simulink model "isi_correlator_tvg"  (Simulink 7.3)
settings: Solver=ode45  StartTime=0.0  StopTime=10.0  RelTol=1e-3
undefined symbols (not defined in the file or in its folder): pfb_coeff_gen_calc, ROACH
libraries: xbsIndex_r4 x5206, casper_library x454, isi_correlator_lib x28, dspsigops x16, xps_library x14
(part 1 of 51)
settings Model: Creator=wjm  LastModifiedBy=wjm

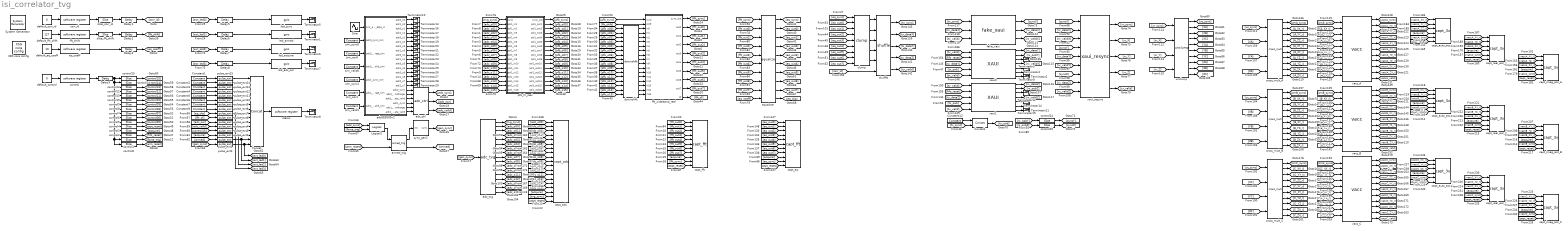
== system isi_correlator_tvg ==
  " System Generator" : xbsIndex_r4/ System Generator  Tag=genX  SystemSampleTime=-1  xilinxfamily=virtex5  part=xc5vsx95t  speed=-1  package=ff1136  synthesis_tool=XST  clock_wrapper=Clock Enables  directory=./isi_correlator_tvg/sysgen  testbench=off  simulink_period=1  sysclk_period=5  dcm_input_clock_period=10  incr_netlist=off  trim_vbits=Everywhere in SubSystem  dbl_ovrd=According to Block Masks  core_generation=According to Block Masks  run_coregen=off  deprecated_control=off  eval_field=0  block_type=sysgen
      settings: block_version=11.3
  Concat : xbsIndex_r4/Concat  num_inputs=16  dbl_ovrd=off  block_type=concat
      settings: block_version=10.1.3
  Concat1 : xbsIndex_r4/Concat  num_inputs=2  dbl_ovrd=off  block_type=concat
      settings: block_version=11.3
  Constant{1..7,9} (8 alike) : xbsIndex_r4/Constant  arith_type=Boolean  const=0  n_bits=1  bin_pt=0  explicit_period=on  period=1  equ=P=C  opselect=C  inp2=PCIN>>17  opr=+  inp1=P  carry=CIN  dbl_ovrd=off  block_type=constant
      settings: block_version=10.1.1
  Constant12 : xbsIndex_r4/Constant  arith_type=Unsigned  const=0  n_bits=3  bin_pt=0  explicit_period=on  period=1  equ=P=C  opselect=C  inp2=PCIN>>17  opr=+  inp1=P  carry=CIN  dbl_ovrd=off  block_type=constant
      settings: block_version=10.1.1
  Delay{1..3,9} (4 alike) : xbsIndex_r4/Delay  en=off  latency=8  dbl_ovrd=off  reg_retiming=off  block_type=delay
  Delay{5..8} (4 alike) : xbsIndex_r4/Delay  en=off  latency=3  dbl_ovrd=off  reg_retiming=off  block_type=delay
  Logical1 : xbsIndex_r4/Logical  logical_function=OR  inputs=2  en=off  latency=1  precision=Full  arith_type=Unsigned  n_bits=1  bin_pt=0  align_bp=on  dbl_ovrd=off  block_type=logical
      settings: block_version=10.1.1
  Sine : Sin  Amplitude=.9  Bias=0  Frequency=37.5*(2*pi/200)  Phase=0  Samples=10  SampleTime=1/16  Offset=0
  Terminator{1..29} (29 alike) : Terminator
  XSG core config : xps_library/XSG core config  Tag=xps:xsg  SystemSampleTime=-1  hw_sys=ROACH:sx95t  ibob_linux=off  clk_src=adc0_clk  iBOB_clk_src=sys_clk  BEE2_ctrl_clk_src=sys_clk  BEE2_usr_clk_src=sys_clk  ROACH_clk_src=adc0_clk  CORR_clk_src=sys_clk  gpio_clk_io_group=iBOB:sma  gpio_clk_bit_index=0  clk_rate=200  sample_period=1  synthesis_tool=XST  mpc_type=powerpc440_ext
  adc083000x2 : Subsystem  Tag=xps:adc083000x2  AncestorBlock=xps_library/adc083000x2  mask(adc_083000x2){use_adc0=on, use_adc1=on, demux_adc0=off, clock_sync=on, verbose=off, adc_clk_rate=800, sample_period=1}
      mask prompts: use_adc0 "Use ZD0K0?"; use_adc1 "Use ZDOK1?"; demux_adc0 "Demux outputs of adc0?"; clock_sync "Interleave 2 ADC boards?"; verbose "Verbose Block Creation?"; adc_clk_rate "ADC clock rate (MHz)"; sample_period "Sample Period"
      mask help: The ADC block converts analog inputs to digital outputs. Every clock cycle, the inputs are sampled and digitized to 8 bit binary point numbers in the range of [-1, 1) and are then output by the adc.
      mask init:
      | adc083000_mask;
      mask Help: eval('xlWeb([getenv(''MLIB_ROOT''), ''\doc\html\sys_adc\sys_adc.html''])')
  adc_ctrl : isi_correlator_lib/adc_ctrl  SystemSampleTime=-1
  adc_tvg : isi_correlator_lib/adc_tvg  SystemSampleTime=-1
  armed_trig : isi_correlator_lib/armed_trig  SystemSampleTime=-1
  capt_adc : isi_correlator_lib/capt_adc  SystemSampleTime=-1
  capt_auto_acc_A : isi_correlator_lib/capt_3x  SystemSampleTime=-1
  capt_auto_acc_B : isi_correlator_lib/capt_3x  SystemSampleTime=-1
  capt_auto_acc_C : isi_correlator_lib/capt_3x  SystemSampleTime=-1
  capt_eq : isi_correlator_lib/capt_fft  SystemSampleTime=-1  bit_width=4
  capt_fft : isi_correlator_lib/capt_fft  SystemSampleTime=-1  bit_width=18
  capt_imag_acc_A : isi_correlator_lib/capt_3x  SystemSampleTime=-1
  capt_imag_acc_B : isi_correlator_lib/capt_3x  SystemSampleTime=-1
  capt_imag_acc_C : isi_correlator_lib/capt_3x  SystemSampleTime=-1
  capt_real_acc_A : isi_correlator_lib/capt_3x  SystemSampleTime=-1
  capt_real_acc_B : isi_correlator_lib/capt_3x  SystemSampleTime=-1
  capt_real_acc_C : isi_correlator_lib/capt_3x  SystemSampleTime=-1
  clump : isi_correlator_lib/clump  SystemSampleTime=-1  latency=1
  control : xps_library/software register  Tag=xps:sw_reg  SystemSampleTime=-1  io_dir=From Processor  arith_type=Unsigned  bitwidth=32  bin_pt=0  sample_period=1  gw_name=isi_correlator_tvg_control_user_data_out
      link override corr_3ghz_10_1_corr_id_user_data_out: arith_type=Unsigned  n_bits=32  bin_pt=0  period=1
      link override corr_3ghz_10_1_exp_corr_id_user_data_out: arith_type=Unsigned  n_bits=32  bin_pt=0  period=1
      link override isi_corr_eq_coeff_user_data_out: arith_type=Unsigned  n_bits=32  bin_pt=0  period=1
      link override isi_correlator_control_user_data_out: arith_type=Unsigned  n_bits=32  bin_pt=0  period=1
      link override isi_correlator_cropped_control_user_data_out: arith_type=Unsigned  n_bits=32  bin_pt=0  period=1
      link override isi_correlator_eq_coeff_user_data_out: arith_type=Unsigned  n_bits=32  bin_pt=0  period=1
      link override isi_spectrometer_control_user_data_out: arith_type=Unsigned  n_bits=32  bin_pt=0  period=1
      link override isi_spectrometer_cropped_control_user_data_out: arith_type=Unsigned  n_bits=32  bin_pt=0  period=1
      link override isi_spectrometer_with_pfb_control_user_data_out: arith_type=Unsigned  n_bits=32  bin_pt=0  period=1
  control0 : xbsIndex_r4/Slice  nbits=1  boolean_output=on  mode=Lower Bit Location + Width  bit1=0  base1=MSB of Input  bit0=0  base0=LSB of Input  dbl_ovrd=off  block_type=slice
      settings: infoedit="Extracts a given range of bits from each input sample and presents it at the output.  The output type is ordinarily unsigned with binary point at zero, but can be Boolean when the slice is one bit wide.<P><P>Hardware notes: In hardware this block costs nothing."  block_version=10.1.3
  control1 : xbsIndex_r4/Slice  nbits=1  boolean_output=on  mode=Lower Bit Location + Width  bit1=0  base1=MSB of Input  bit0=1  base0=LSB of Input  dbl_ovrd=off  block_type=slice
      settings: infoedit="Extracts a given range of bits from each input sample and presents it at the output.  The output type is ordinarily unsigned with binary point at zero, but can be Boolean when the slice is one bit wide.<P><P>Hardware notes: In hardware this block costs nothing."  block_version=10.1.3
  control10 : xbsIndex_r4/Slice  nbits=1  boolean_output=on  mode=Lower Bit Location + Width  bit1=0  base1=MSB of Input  bit0=10  base0=LSB of Input  dbl_ovrd=off  block_type=slice
      settings: infoedit="Extracts a given range of bits from each input sample and presents it at the output.  The output type is ordinarily unsigned with binary point at zero, but can be Boolean when the slice is one bit wide.<P><P>Hardware notes: In hardware this block costs nothing."  block_version=10.1.3
  control11 : xbsIndex_r4/Slice  nbits=1  boolean_output=on  mode=Lower Bit Location + Width  bit1=0  base1=MSB of Input  bit0=11  base0=LSB of Input  dbl_ovrd=off  block_type=slice
      settings: infoedit="Extracts a given range of bits from each input sample and presents it at the output.  The output type is ordinarily unsigned with binary point at zero, but can be Boolean when the slice is one bit wide.<P><P>Hardware notes: In hardware this block costs nothing."  block_version=10.1.3
  control12 : xbsIndex_r4/Slice  nbits=1  boolean_output=on  mode=Lower Bit Location + Width  bit1=0  base1=MSB of Input  bit0=12  base0=LSB of Input  dbl_ovrd=off  block_type=slice
      settings: infoedit="Extracts a given range of bits from each input sample and presents it at the output.  The output type is ordinarily unsigned with binary point at zero, but can be Boolean when the slice is one bit wide.<P><P>Hardware notes: In hardware this block costs nothing."  block_version=10.1.3
  control13 : xbsIndex_r4/Slice  nbits=1  boolean_output=on  mode=Lower Bit Location + Width  bit1=0  base1=MSB of Input  bit0=13  base0=LSB of Input  dbl_ovrd=off  block_type=slice
      settings: infoedit="Extracts a given range of bits from each input sample and presents it at the output.  The output type is ordinarily unsigned with binary point at zero, but can be Boolean when the slice is one bit wide.<P><P>Hardware notes: In hardware this block costs nothing."  block_version=10.1.3
  control14 : xbsIndex_r4/Slice  nbits=1  boolean_output=on  mode=Lower Bit Location + Width  bit1=0  base1=MSB of Input  bit0=14  base0=LSB of Input  dbl_ovrd=off  block_type=slice
      settings: infoedit="Extracts a given range of bits from each input sample and presents it at the output.  The output type is ordinarily unsigned with binary point at zero, but can be Boolean when the slice is one bit wide.<P><P>Hardware notes: In hardware this block costs nothing."  block_version=10.1.3
  control15 : xbsIndex_r4/Slice  nbits=1  boolean_output=on  mode=Lower Bit Location + Width  bit1=0  base1=MSB of Input  bit0=15  base0=LSB of Input  dbl_ovrd=off  block_type=slice
      settings: infoedit="Extracts a given range of bits from each input sample and presents it at the output.  The output type is ordinarily unsigned with binary point at zero, but can be Boolean when the slice is one bit wide.<P><P>Hardware notes: In hardware this block costs nothing."  block_version=10.1.3
  control2 : xbsIndex_r4/Slice  nbits=1  boolean_output=on  mode=Lower Bit Location + Width  bit1=0  base1=MSB of Input  bit0=2  base0=LSB of Input  dbl_ovrd=off  block_type=slice
      settings: infoedit="Extracts a given range of bits from each input sample and presents it at the output.  The output type is ordinarily unsigned with binary point at zero, but can be Boolean when the slice is one bit wide.<P><P>Hardware notes: In hardware this block costs nothing."  block_version=10.1.3
  control24 : xbsIndex_r4/Slice  nbits=1  boolean_output=on  mode=Lower Bit Location + Width  bit1=0  base1=MSB of Input  bit0=0  base0=LSB of Input  dbl_ovrd=off  block_type=slice
      settings: infoedit="Extracts a given range of bits from each input sample and presents it at the output.  The output type is ordinarily unsigned with binary point at zero, but can be Boolean when the slice is one bit wide.<P><P>Hardware notes: In hardware this block costs nothing."  block_version=10.1.3
  control25 : xbsIndex_r4/Slice  nbits=1  boolean_output=on  mode=Lower Bit Location + Width  bit1=0  base1=MSB of Input  bit0=0  base0=LSB of Input  dbl_ovrd=off  block_type=slice
      settings: infoedit="Extracts a given range of bits from each input sample and presents it at the output.  The output type is ordinarily unsigned with binary point at zero, but can be Boolean when the slice is one bit wide.<P><P>Hardware notes: In hardware this block costs nothing."  block_version=10.1.3
  control3 : xbsIndex_r4/Slice  nbits=1  boolean_output=on  mode=Lower Bit Location + Width  bit1=0  base1=MSB of Input  bit0=3  base0=LSB of Input  dbl_ovrd=off  block_type=slice
      settings: infoedit="Extracts a given range of bits from each input sample and presents it at the output.  The output type is ordinarily unsigned with binary point at zero, but can be Boolean when the slice is one bit wide.<P><P>Hardware notes: In hardware this block costs nothing."  block_version=10.1.3
  control4 : xbsIndex_r4/Slice  nbits=1  boolean_output=on  mode=Lower Bit Location + Width  bit1=0  base1=MSB of Input  bit0=4  base0=LSB of Input  dbl_ovrd=off  block_type=slice
      settings: infoedit="Extracts a given range of bits from each input sample and presents it at the output.  The output type is ordinarily unsigned with binary point at zero, but can be Boolean when the slice is one bit wide.<P><P>Hardware notes: In hardware this block costs nothing."  block_version=10.1.3
  control5 : xbsIndex_r4/Slice  nbits=1  boolean_output=on  mode=Lower Bit Location + Width  bit1=0  base1=MSB of Input  bit0=5  base0=LSB of Input  dbl_ovrd=off  block_type=slice
      settings: infoedit="Extracts a given range of bits from each input sample and presents it at the output.  The output type is ordinarily unsigned with binary point at zero, but can be Boolean when the slice is one bit wide.<P><P>Hardware notes: In hardware this block costs nothing."  block_version=10.1.3
  control6 : xbsIndex_r4/Slice  nbits=1  boolean_output=on  mode=Lower Bit Location + Width  bit1=0  base1=MSB of Input  bit0=6  base0=LSB of Input  dbl_ovrd=off  block_type=slice
      settings: infoedit="Extracts a given range of bits from each input sample and presents it at the output.  The output type is ordinarily unsigned with binary point at zero, but can be Boolean when the slice is one bit wide.<P><P>Hardware notes: In hardware this block costs nothing."  block_version=10.1.3
  control7 : xbsIndex_r4/Slice  nbits=1  boolean_output=on  mode=Lower Bit Location + Width  bit1=0  base1=MSB of Input  bit0=7  base0=LSB of Input  dbl_ovrd=off  block_type=slice
      settings: infoedit="Extracts a given range of bits from each input sample and presents it at the output.  The output type is ordinarily unsigned with binary point at zero, but can be Boolean when the slice is one bit wide.<P><P>Hardware notes: In hardware this block costs nothing."  block_version=10.1.3
  control8 : xbsIndex_r4/Slice  nbits=1  boolean_output=on  mode=Lower Bit Location + Width  bit1=0  base1=MSB of Input  bit0=8  base0=LSB of Input  dbl_ovrd=off  block_type=slice
      settings: infoedit="Extracts a given range of bits from each input sample and presents it at the output.  The output type is ordinarily unsigned with binary point at zero, but can be Boolean when the slice is one bit wide.<P><P>Hardware notes: In hardware this block costs nothing."  block_version=10.1.3
  control9 : xbsIndex_r4/Slice  nbits=1  boolean_output=on  mode=Lower Bit Location + Width  bit1=0  base1=MSB of Input  bit0=9  base0=LSB of Input  dbl_ovrd=off  block_type=slice
      settings: infoedit="Extracts a given range of bits from each input sample and presents it at the output.  The output type is ordinarily unsigned with binary point at zero, but can be Boolean when the slice is one bit wide.<P><P>Hardware notes: In hardware this block costs nothing."  block_version=10.1.3
  corr_id : xps_library/software register  Tag=xps:sw_reg  SystemSampleTime=-1  io_dir=From Processor  arith_type=Unsigned  bitwidth=32  bin_pt=0  sample_period=1  gw_name=isi_correlator_tvg_corr_id_user_data_out
      link override corr_3ghz_10_1_corr_id_user_data_out: arith_type=Unsigned  n_bits=32  bin_pt=0  period=1
      link override corr_3ghz_10_1_exp_corr_id_user_data_out: arith_type=Unsigned  n_bits=32  bin_pt=0  period=1
      link override isi_corr_corr_id_user_data_out: arith_type=Unsigned  n_bits=32  bin_pt=0  period=1
      link override isi_correlator_corr_id_user_data_out: arith_type=Unsigned  n_bits=32  bin_pt=0  period=1
      link override isi_correlator_cropped_corr_id_user_data_out: arith_type=Unsigned  n_bits=32  bin_pt=0  period=1
      link override isi_spectrometer_corr_id_user_data_out: arith_type=Unsigned  n_bits=32  bin_pt=0  period=1
      link override isi_spectrometer_cropped_corr_id_user_data_out: arith_type=Unsigned  n_bits=32  bin_pt=0  period=1
      link override isi_spectrometer_with_pfb_corr_id_user_data_out: arith_type=Unsigned  n_bits=32  bin_pt=0  period=1
  cross_mult_A : isi_correlator_lib/cross_mult  SystemSampleTime=-1  in_bit_width=4  in_bin_point=3  out_bit_width=8  out_bin_point=6  mult_latency=3  add_latency=2
  cross_mult_B : isi_correlator_lib/cross_mult  SystemSampleTime=-1  in_bit_width=4  in_bin_point=3  out_bit_width=8  out_bin_point=6  mult_latency=3  add_latency=2
  cross_mult_C : isi_correlator_lib/cross_mult  SystemSampleTime=-1  in_bit_width=4  in_bin_point=3  out_bit_width=8  out_bin_point=6  mult_latency=3  add_latency=2
  default_control : Constant  Value=0  OutDataType=sfix(16)  OutScaling=2^0  SampleTime=1
  default_corr_id : Constant  Value=0  OutDataType=sfix(16)  OutScaling=2^0  SampleTime=1
  default_eq_coeff : Constant  Value=10  OutDataType=sfix(16)  OutScaling=2^0  SampleTime=1
  default_fft_shift : Constant  Value=127  OutDataType=sfix(16)  OutScaling=2^0  SampleTime=1
  downshift : isi_correlator_lib/downshift  SystemSampleTime=-1  bit_width=18
  eq_coeff : xps_library/software register  Tag=xps:sw_reg  SystemSampleTime=-1  io_dir=From Processor  arith_type=Unsigned  bitwidth=32  bin_pt=0  sample_period=1  gw_name=isi_correlator_tvg_eq_coeff_user_data_out
      link override corr_3ghz_10_1_corr_id_user_data_out: arith_type=Unsigned  n_bits=32  bin_pt=0  period=1
      link override corr_3ghz_10_1_exp_corr_id_user_data_out: arith_type=Unsigned  n_bits=32  bin_pt=0  period=1
      link override isi_corr_eq_coeff_user_data_out: arith_type=Unsigned  n_bits=32  bin_pt=0  period=1
      link override isi_correlator_cropped_eq_coeff_user_data_out: arith_type=Unsigned  n_bits=32  bin_pt=0  period=1
      link override isi_correlator_eq_coeff_user_data_out: arith_type=Unsigned  n_bits=32  bin_pt=0  period=1
      link override isi_spectrometer_cropped_eq_coeff_user_data_out: arith_type=Unsigned  n_bits=32  bin_pt=0  period=1
      link override isi_spectrometer_eq_coeff_user_data_out: arith_type=Unsigned  n_bits=32  bin_pt=0  period=1
      link override isi_spectrometer_with_pfb_eq_coeff_user_data_out: arith_type=Unsigned  n_bits=32  bin_pt=0  period=1
  equalizer : isi_correlator_lib/equalizer  SystemSampleTime=-1  in_bit_width=18  in_bin_point=17  coeff_bit_width=16  coeff_bin_point=8  out_bit_width=4  out_bin_point=3
  fake_xaui : isi_correlator_lib/fake_xaui  SystemSampleTime=-1  latency=32
  fft_shift : xps_library/software register  Tag=xps:sw_reg  SystemSampleTime=-1  io_dir=From Processor  arith_type=Unsigned  bitwidth=32  bin_pt=0  sample_period=1  gw_name=isi_correlator_tvg_fft_shift_user_data_out
      link override corr_3ghz_10_1_corr_id_user_data_out: arith_type=Unsigned  n_bits=32  bin_pt=0  period=1
      link override corr_3ghz_10_1_exp_corr_id_user_data_out: arith_type=Unsigned  n_bits=32  bin_pt=0  period=1
      link override isi_corr_eq_coeff_user_data_out: arith_type=Unsigned  n_bits=32  bin_pt=0  period=1
      link override isi_correlator_cropped_eq_coeff_user_data_out: arith_type=Unsigned  n_bits=32  bin_pt=0  period=1
      link override isi_correlator_eq_coeff_user_data_out: arith_type=Unsigned  n_bits=32  bin_pt=0  period=1
      link override isi_spectrometer_cropped_eq_coeff_user_data_out: arith_type=Unsigned  n_bits=32  bin_pt=0  period=1
      link override isi_spectrometer_fft_shift_user_data_out: arith_type=Unsigned  n_bits=32  bin_pt=0  period=1
      link override isi_spectrometer_with_pfb_eq_coeff_user_data_out: arith_type=Unsigned  n_bits=32  bin_pt=0  period=1
  fft_wideband_real : Subsystem  AncestorBlock=casper_library/FFTs/fft_wideband_real  mask(fft_wideband_real){FFTSize=7, input_bit_width=18, coeff_bit_width=18, n_inputs=4, quantization=Truncate, overflow=Wrap, add_latency=1, mult_latency=3, bram_latency=3, arch=Virtex5, opt_target=Multipliers, coeffs_bit_limit=8, delays_bit_limit=9, specify_mult=on, mult_spec=[1 1 1 1 1 1 1], dsp48_adders=on}
      mask prompts: FFTSize "Size of FFT: (2^?)"; input_bit_width "Input/Output Bit Width"; coeff_bit_width "Coefficient Bit Width"; n_inputs "Number of Simultaneous Inputs: (2^?)"; quantization "Quantization Behavior"; overflow "Overflow Behavior"; add_latency "Add Latency"; mult_latency "Mult Latency"; bram_latency "BRAM Latency"; arch "Architecture"; opt_target "Optimisation Target"; coeffs_bit_limit "Number bits above which to store stage's coefficients in BRAM (2^? bits)"; delays_bit_limit "Number bits above which to store stage's delays in BRAM (2^? bits)"; specify_mult "Specify multiplier use (behavioral HDL if not specified)"; mult_spec "Multiplier specification (0=core, 1=embedded, 2=behavioural) (left=1st stage)"; dsp48_adders "Use DSP48s for adders"
      mask init:
      | fft_wideband_real_init(gcb,...
      |     'FFTSize', FFTSize,...
      |     'input_bit_width', input_bit_width,...
      |     'coeff_bit_width', coeff_bit_width,...
      |     'n_inputs', n_inputs,...
      |     'quantization', quantization,...
      |     'overflow', overflow,...
      |     'add_latency', add_latency,...
      |     'mult_latency', mult_latency,...
      |     'bram_latency', bram_latency,...
      |     'arch', arch, ...
      |     'opt_target', opt_target, ...
      |     'coeffs_bit_limit', coeffs_bit_limit, ...
      |     'delays_bit_limit', delays_bit_limit, ...    
      |     'specify_mult', specify_mult,...
      |     'mult_spec', mult_spec, ...
      |     'dsp48_adders', dsp48_adders);
      settings: AttributesFormatString=FFTSize=7
      mask Help: eval('xlWeb([getenv(''MLIB_ROOT''), ''\doc\htmlblocks\sp_fft_wideband_real\sp_fft_wideband_real.html''])')
      mask StyleString: edit,edit,edit,edit,popup(Truncate|Round  (unbiased: +/- Inf)|Round  (unbiased: Even Values)),popup(Wrap|Saturate|Error),edit,edit,edit,popup(Virtex2Pro|Virtex5),popup(Logic|Multipliers),edit,edit,checkbox,edit,checkbox
      mask CallbackString:
      | % fft_size = eval(get_param(gcb, 'FFTSize'));
      | % old_vec = eval(get_param(gcb, 'mult_spec'));
      | 
      | % vec = 2.*ones(1,fft_size);
      | % old_size = length(old_vec);
      | 
      | % if(old_size <= fft_size),
      | %    size = old_size;
      | % else,
      | %     size = fft_size;
      | % end
      | 
      | % for n = 1:size, vec(n) = old_vec(n); end
      | % set_param(gcb, 'mult_spec', mat2str(vec));|||||||||||||specify_mult = get_param(gcb,'specify_mult');
      | visibs = get_param(gcb, 'MaskVisibilities');
      | enables = get_param(gcb, 'MaskEnables');
      | enables{15} = 'on';
      | if( strcmp(specify_mult,'off')), visibs{15} = 'off';
      | else, visibs{15} = 'on';
      | end
      | set_param(gcb, 'MaskEnables', enables);
      | set_param(gcb, 'MaskVisibilities', visibs);
      | |% vec = eval( get_param(gcb, 'mult_spec'));
      | % vec_size = length(vec);
      | % fft_size = eval( get_param(gcb, 'FFTSize'));
      | % new_vec = 2.*ones(1, fft_size);
      | 
      | % if(vec_size <= fft_size), size = vec_size;
      | % else size = fft_size;
      | % end
      | 
      | % for n=1:size
      | %     new_vec(n) = vec(n);
      | % end
      | % set_param(gcb, 'mult_spec', mat2str(new_vec));|
  led_acquire : xps_library/gpio  Tag=xps:gpio  SystemSampleTime=-1  io_group=ROACH:led  io_dir=out  arith_type=Boolean  bitwidth=1  bin_pt=0  bit_index=2  sample_period=1  use_single_ended=off  use_ddr=off  reg_iob=on  reg_clk_phase=0  termination=None
      link override convert: arith_type=Boolean  n_bits=1  bin_pt=0
  led_adc_oor : xps_library/gpio  Tag=xps:gpio  SystemSampleTime=-1  io_group=ROACH:led  io_dir=out  arith_type=Boolean  bitwidth=1  bin_pt=0  bit_index=3  sample_period=1  use_single_ended=off  use_ddr=off  reg_iob=on  reg_clk_phase=0  termination=None
      link override convert: arith_type=Boolean  n_bits=1  bin_pt=0
  led_armed : xps_library/gpio  Tag=xps:gpio  SystemSampleTime=-1  io_group=ROACH:led  io_dir=out  arith_type=Boolean  bitwidth=1  bin_pt=0  bit_index=1  sample_period=1  use_single_ended=off  use_ddr=off  reg_iob=on  reg_clk_phase=0  termination=None
      link override convert: arith_type=Boolean  n_bits=1  bin_pt=0
  led_sync : xps_library/gpio  Tag=xps:gpio  SystemSampleTime=-1  io_group=ROACH:led  io_dir=out  arith_type=Boolean  bitwidth=1  bin_pt=0  bit_index=0  sample_period=1  use_single_ended=off  use_ddr=off  reg_iob=on  reg_clk_phase=0  termination=None
      link override convert: arith_type=Boolean  n_bits=1  bin_pt=0
  pfb_fir_real : Subsystem  AncestorBlock=casper_library/PFBs/pfb_fir_real  mask(pfb_fir_real){PFBSize=7, TotalTaps=4, WindowType=hamming, n_inputs=4, MakeBiplex=0, BitWidthIn=8, BitWidthOut=18, CoeffBitWidth=18, CoeffDistMem=0, add_latency=2, mult_latency=3, bram_latency=2, quantization=Truncate, fwidth=1, specify_mult=on, mult_spec=[1 1 1 1], behavioral=off}
      mask prompts: PFBSize "Size of PFB: (2^? pnts)"; TotalTaps "Total Number of Taps:"; WindowType "Windowing Function:"; n_inputs "Number of Simultaneous Inputs: (2^?)"; MakeBiplex "Make Biplex"; BitWidthIn "Input Bitwidth:"; BitWidthOut "Output Bitwidth:"; CoeffBitWidth "Coefficient Bitwidth:"; CoeffDistMem "Use Distributed Memory for Coeffs"; add_latency "Add Latency"; mult_latency "Mult Latency"; bram_latency "BRAM Latency"; quantization "Quantization Behavior"; fwidth "Bin Width Scaling (normal=1)"; specify_mult "Specify multiplier use (embedded if not specified)"; mult_spec "Multiplier specification (0=core, 1=embedded, 2=behavioural) (left=1st tap)"; behavioral "Use behavioral HDL for adder trees"
      mask init:
      | pfb_fir_real_init(gcb,...
      |     'PFBSize', PFBSize,...
      |     'TotalTaps', TotalTaps,...
      |     'WindowType', WindowType,...
      |     'n_inputs', n_inputs,...
      |     'MakeBiplex', MakeBiplex,...
      |     'BitWidthIn', BitWidthIn,...
      |     'BitWidthOut', BitWidthOut,...
      |     'CoeffBitWidth', CoeffBitWidth,...
      |     'CoeffDistMem', CoeffDistMem,...
      |     'add_latency', add_latency,...
      |     'mult_latency', mult_latency,...
      |     'bram_latency', bram_latency,...
      |     'quantization', quantization,...
      |     'fwidth', fwidth,...
      |     'specify_mult', specify_mult,...
      |     'mult_spec', mult_spec,...
      |     'behavioral', behavioral);
      settings: AttributesFormatString=taps=4, add_latency=2
      mask Help: eval('xlWeb([getenv(''MLIB_ROOT''), ''\doc\htmlblocks\sp_pfb_fir_real\sp_pfb_fir_real.html''])')
      mask StyleString: edit,edit,popup(bartlett|barthannwin|blackman|blackmanharris|bohamwin|chebwin|flattopwin|gausswin|hamming|hann|kaiser|nuttallwin|parzenwin|rectwin|tukeywin|triang|userwindow),edit,edit,edit,edit,edit,edit,edit,edit,edit,popup(Truncate|Round  (unbiased: +/- Inf)|Round  (unbiased: Even Values)),edit,checkbox,edit,checkbox
      mask CallbackString:
      | |num_taps = eval(get_param(gcb, 'TotalTaps'));
      | old_vec = eval(get_param(gcb, 'mult_spec'));
      | 
      | vec = ones(1,num_taps);
      | old_size = length(old_vec);
      | 
      | if(old_size <= num_taps),
      |     size = old_size;
      | else,
      |     size = num_taps;
      | end
      | 
      | for n = 1:size, vec(n) = old_vec(n); end
      | set_param(gcb, 'mult_spec', mat2str(vec));|||||||||||||thisVariable = 'specify_mult';
      | affectedVars = {'mult_spec'};
      | 
      | thisBlock = gcb();
      | thisVarState = get_param(thisBlock, thisVariable);
      | maskNames = get_param(thisBlock, 'MaskNames');
      | maskEnables = get_param(thisBlock, 'MaskEnables');
      | 
      | for varName = affectedVars
      |     varIndex = find(ismember(maskNames, varName));
      |     maskEnables{varIndex} = thisVarState;
      | end
      | 
      | set_param(thisBlock, 'MaskEnables', maskEnables);|vec = eval( get_param(gcb, 'mult_spec'));
      | vec_size = length(vec);
      | num_taps = eval( get_param(gcb, 'TotalTaps'));
      | new_vec = ones(1, num_taps);
      | 
      | if(vec_size <= num_taps), size = vec_size;
      | else size = num_taps;
      | end
      | 
      | for n=1:size
      |     new_vec(n) = vec(n);
      | end
      | set_param(gcb, 'mult_spec', mat2str(new_vec));|
  pulse_ext{0..15} (16 alike) : casper_library/Misc/pulse_ext  SystemSampleTime=-1  pulse_len=5
  shuffle : isi_correlator_lib/shuffle  SystemSampleTime=-1  latency=3
  sim_oor0 : xbsIndex_r4/Constant  arith_type=Unsigned  const=0  n_bits=4  bin_pt=0  explicit_period=on  period=1  equ=P=C  opselect=C  inp2=PCIN>>17  opr=+  inp1=P  carry=CIN  dbl_ovrd=off  block_type=constant
      settings: block_version=10.1.1
  sim_oor1 : xbsIndex_r4/Constant  arith_type=Unsigned  const=0  n_bits=4  bin_pt=0  explicit_period=on  period=1  equ=P=C  opselect=C  inp2=PCIN>>17  opr=+  inp1=P  carry=CIN  dbl_ovrd=off  block_type=constant
      settings: block_version=10.1.1
  sim_sync0 : xbsIndex_r4/Constant  arith_type=Unsigned  const=0  n_bits=4  bin_pt=0  explicit_period=on  period=1  equ=P=C  opselect=C  inp2=PCIN>>17  opr=+  inp1=P  carry=CIN  dbl_ovrd=off  block_type=constant
      settings: block_version=10.1.1
  sim_sync1 : xbsIndex_r4/Constant  arith_type=Unsigned  const=0  n_bits=4  bin_pt=0  explicit_period=on  period=1  equ=P=C  opselect=C  inp2=PCIN>>17  opr=+  inp1=P  carry=CIN  dbl_ovrd=off  block_type=constant
      settings: block_version=10.1.1
  sim_valid0 : xbsIndex_r4/Constant  arith_type=Unsigned  const=0  n_bits=1  bin_pt=0  explicit_period=on  period=1  equ=P=C  opselect=C  inp2=PCIN>>17  opr=+  inp1=P  carry=CIN  dbl_ovrd=off  block_type=constant
      settings: block_version=10.1.1
  sim_valid1 : xbsIndex_r4/Constant  arith_type=Unsigned  const=0  n_bits=1  bin_pt=0  explicit_period=on  period=1  equ=P=C  opselect=C  inp2=PCIN>>17  opr=+  inp1=P  carry=CIN  dbl_ovrd=off  block_type=constant
      settings: block_version=10.1.1
  slice_corr_id : xbsIndex_r4/Slice  nbits=2  boolean_output=off  mode=Lower Bit Location + Width  bit1=0  base1=MSB of Input  bit0=0  base0=LSB of Input  dbl_ovrd=off  block_type=slice
      settings: infoedit="Extracts a given range of bits from each input sample and presents it at the output.  The output type is ordinarily unsigned with binary point at zero, but can be Boolean when the slice is one bit wide.<P><P>Hardware notes: In hardware this block costs nothing."  block_version=10.1.3
  slice_fft_shift : xbsIndex_r4/Slice  nbits=7  boolean_output=off  mode=Lower Bit Location + Width  bit1=0  base1=MSB of Input  bit0=0  base0=LSB of Input  dbl_ovrd=off  block_type=slice
      settings: infoedit="Extracts a given range of bits from each input sample and presents it at the output.  The output type is ordinarily unsigned with binary point at zero, but can be Boolean when the slice is one bit wide.<P><P>Hardware notes: In hardware this block costs nothing."  block_version=10.1.3
  status : xps_library/software register  Tag=xps:sw_reg  SystemSampleTime=-1  io_dir=To Processor  arith_type=Unsigned  bitwidth=32  bin_pt=0  sample_period=1  gw_name=isi_correlator_tvg_status_user_data_in
      link override convert: arith_type=Unsigned  n_bits=32  bin_pt=0
      link override corr_3ghz_10_1_corr_id_user_data_out: arith_type=Unsigned  n_bits=32  bin_pt=0  period=1
      link override corr_3ghz_10_1_exp_corr_id_user_data_out: arith_type=Unsigned  n_bits=32  bin_pt=0  period=1
      link override isi_corr_eq_coeff_user_data_out: arith_type=Unsigned  n_bits=32  bin_pt=0  period=1
      link override isi_correlator_eq_coeff_user_data_out: arith_type=Unsigned  n_bits=32  bin_pt=0  period=1
  sync_gen2 : Subsystem  AncestorBlock=isi_correlator_lib/sync_gen2  mask(sync_gen2){using_pfb_fir=on, pfb_fir_taps=4, using_fft=on, fft_size=7, fft_inputs=4, fft_orders=[2 2 2 1], using_ct=off, ct_type=Double-buffered, using_vacc=off, vacc_length=0, using_scale_factor=off, scale_factor=1}
      mask prompts: using_pfb_fir "This design uses a PFB FIR."; pfb_fir_taps "Number of taps in PFB FIR:"; using_fft "This design uses an FFT."; fft_size "Size of FFT: (2^?)"; fft_inputs "Number of FFT inputs: (2^?)"; fft_orders "Order of FFT reorders: (vector)"; using_ct "This design uses a corner turner."; ct_type "Type of reorder operation:"; using_vacc "This design uses a vector accumulator."; vacc_length "Length of accumulations: (2^?)"; using_scale_factor "Scale the minimum period by an arbitrary amount."; scale_factor "Scale factor:"
      mask help: This block generates a sync pulse of an appropriate period based on the specified mask parameters.
      mask init:
      | sync_gen2_init(gcb, ...
      |     'using_pfb_fir', using_pfb_fir, ...
      |     'pfb_fir_taps', pfb_fir_taps, ...
      |     'using_fft', using_fft, ...
      |     'fft_size', fft_size, ...
      |     'fft_inputs', fft_inputs, ...
      |     'fft_orders', fft_orders, ...
      |     'using_ct', using_ct, ...
      |     'ct_type', ct_type, ...
      |     'using_vacc', using_vacc, ...
      |     'vacc_length', vacc_length, ...
      |     'using_scale_factor', using_scale_factor, ...
      |     'scale_factor', scale_factor);
      settings: AttributesFormatString=sync_period=16
      mask StyleString: checkbox,edit,checkbox,edit,edit,edit,checkbox,popup(Double-buffered|In-place),checkbox,edit,checkbox,edit
      mask CallbackString:
      | thisVariable = 'using_pfb_fir';
      | affectedVars = {'pfb_fir_taps'};
      | 
      | thisBlock = gcb();
      | thisVarState = get_param(thisBlock, thisVariable);
      | maskNames = get_param(thisBlock, 'MaskNames');
      | maskEnables = get_param(thisBlock, 'MaskEnables');
      | 
      | for varName = affectedVars
      |     varIndex = find(ismember(maskNames, varName));
      |     maskEnables{varIndex} = thisVarState;
      | end
      | 
      | set_param(thisBlock, 'MaskEnables', maskEnables);||thisVariable = 'using_fft';
      | affectedVars = {'fft_size', 'fft_inputs', 'fft_orders'};
      | 
      | thisBlock = gcb();
      | thisVarState = get_param(thisBlock, thisVariable);
      | maskNames = get_param(thisBlock, 'MaskNames');
      | maskEnables = get_param(thisBlock, 'MaskEnables');
      | 
      | for varName = affectedVars
      |     varIndex = find(ismember(maskNames, varName));
      |     maskEnables{varIndex} = thisVarState;
      | end
      | 
      | set_param(thisBlock, 'MaskEnables', maskEnables);||||thisVariable = 'using_ct';
      | affectedVars = {'ct_type'};
      | 
      | thisBlock = gcb();
      | thisVarState = get_param(thisBlock, thisVariable);
      | maskNames = get_param(thisBlock, 'MaskNames');
      | maskEnables = get_param(thisBlock, 'MaskEnables');
      | 
      | for varName = affectedVars
      |     varIndex = find(ismember(maskNames, varName));
      |     maskEnables{varIndex} = thisVarState;
      | end
      | 
      | set_param(thisBlock, 'MaskEnables', maskEnables);||thisVariable = 'using_vacc';
      | affectedVars = {'vacc_length'};
      | 
      | thisBlock = gcb();
      | thisVarState = get_param(thisBlock, thisVariable);
      | maskNames = get_param(thisBlock, 'MaskNames');
      | maskEnables = get_param(thisBlock, 'MaskEnables');
      | 
      | for varName = affectedVars
      |     varIndex = find(ismember(maskNames, varName));
      |     maskEnables{varIndex} = thisVarState;
      | end
      | 
      | set_param(thisBlock, 'MaskEnables', maskEnables);||thisVariable = 'using_scale_factor';
      | affectedVars = {'scale_factor'};
      | 
      | thisBlock = gcb();
      | thisVarState = get_param(thisBlock, thisVariable);
      | maskNames = get_param(thisBlock, 'MaskNames');
      | maskEnables = get_param(thisBlock, 'MaskEnables');
      | 
      | for varName = affectedVars
      |     varIndex = find(ismember(maskNames, varName));
      |     maskEnables{varIndex} = thisVarState;
      | end
      | 
      | set_param(thisBlock, 'MaskEnables', maskEnables);|
  unclump : isi_correlator_lib/unclump  SystemSampleTime=-1  latency=1
  vacc_A : isi_correlator_lib/vacc  SystemSampleTime=-1  vector_length=8  out_bit_width=27  out_bin_point=4  and_delay=2
  vacc_B : isi_correlator_lib/vacc  SystemSampleTime=-1  vector_length=8  out_bit_width=27  out_bin_point=4  and_delay=2
  vacc_C : isi_correlator_lib/vacc  SystemSampleTime=-1  vector_length=8  out_bit_width=27  out_bin_point=4  and_delay=2
  xaui0 : xps_library/XAUI  Tag=xps:xaui  SystemSampleTime=-1  demux=2  port=ROACH:0  open_phy=on  show_param=off  pre_emph=3  swing=800
      link override convert_tx_data: n_bits=32
      link override convert_tx_outofband: n_bits=4
      link override test_link_mux_demux_xaui0_rx_data: n_bits=32
      link override test_link_mux_demux_xaui0_rx_outofband: n_bits=4
  xaui1 : xps_library/XAUI  Tag=xps:xaui  SystemSampleTime=-1  demux=2  port=ROACH:1  open_phy=on  show_param=off  pre_emph=3  swing=800
      link override convert_tx_data: n_bits=32
      link override convert_tx_outofband: n_bits=4
      link override test_link_mux_demux_xaui1_rx_data: n_bits=32
      link override test_link_mux_demux_xaui1_rx_outofband: n_bits=4
  xaui_resync : isi_correlator_lib/xaui_resync  SystemSampleTime=-1  buffer_depth=8  logic_latency=1
  wires:
  [pfb_sync] -> fft_wideband_real/1(sync)
  default_eq_coeff/1 -> eq_coeff/1
  default_control/1 -> control/1
  led_sync/1 -> Terminator5/1
  led_armed/1 -> Terminator1/1
  led_acquire/1 -> Terminator6/1
  led_adc_oor/1 -> Terminator7/1
  [ext_led0] -> Delay5/1
  eq_coeff/1 -> Delay3/1
  [ext_led1] -> Delay6/1
  [ext_led2] -> Delay7/1
  [control15] -> pulse_ext3/1
  [acquire] -> pulse_ext2/1
  [armed] -> pulse_ext1/1
  [gen_sync] -> pulse_ext0/1
  Constant1/1 -> pulse_ext15/1
  Constant2/1 -> pulse_ext14/1
  Constant3/1 -> pulse_ext13/1
  Constant4/1 -> pulse_ext12/1
  Constant5/1 -> pulse_ext11/1
  Constant6/1 -> pulse_ext10/1
  Constant7/1 -> pulse_ext9/1
  Constant9/1 -> pulse_ext8/1
  [eq_clip] -> pulse_ext7/1
  [fft_of] -> pulse_ext6/1
  [adc_oor] -> pulse_ext5/1
  [adc_valid] -> pulse_ext4/1
  pulse_ext15/1 -> Concat/1
  pulse_ext14/1 -> Concat/2
  pulse_ext13/1 -> Concat/3
  pulse_ext12/1 -> Concat/4
  pulse_ext11/1 -> Concat/5
  pulse_ext10/1 -> Concat/6
  pulse_ext9/1 -> Concat/7
  pulse_ext8/1 -> Concat/8
  pulse_ext7/1 -> Concat/9, [ext_led3]
  pulse_ext6/1 -> Concat/10
  pulse_ext5/1 -> Concat/11
  pulse_ext4/1 -> Concat/12
  Sine/1 -> adc083000x2/1(sim_adc_data_in)
  fft_wideband_real/2(out0) -> [fft_out0]
  fft_wideband_real/3(out1) -> [fft_out1]
  fft_wideband_real/4(out2) -> [fft_out2]
  fft_wideband_real/5(out3) -> [fft_out3]
  fft_wideband_real/6(out4) -> [fft_out4]
  fft_wideband_real/7(out5) -> [fft_out5]
  fft_wideband_real/8(out6) -> [fft_out6]
  fft_wideband_real/9(out7) -> [fft_out7]
  [fft_out0] -> capt_fft/1
  [fft_out1] -> capt_fft/2
  [fft_out2] -> capt_fft/3
  [fft_out3] -> capt_fft/4
  [fft_out4] -> capt_fft/5
  [fft_out5] -> capt_fft/6
  [fft_out6] -> capt_fft/7
  [fft_out7] -> capt_fft/8
  [tvg_sync] -> pfb_fir_real/1(sync)
  [adc_o00] -> pfb_fir_real/2(pol1_in1)
  [adc_o01] -> pfb_fir_real/3(pol1_in2)
  [adc_o02] -> pfb_fir_real/4(pol1_in3)
  [adc_o03] -> pfb_fir_real/5(pol1_in4)
  [adc_o04] -> pfb_fir_real/6(pol1_in5)
  [adc_o05] -> pfb_fir_real/7(pol1_in6)
  [adc_o06] -> pfb_fir_real/8(pol1_in7)
  [adc_o07] -> pfb_fir_real/9(pol1_in8)
  [adc_o08] -> pfb_fir_real/10(pol1_in9)
  [adc_o09] -> pfb_fir_real/11(pol1_in10)
  [adc_o10] -> pfb_fir_real/12(pol1_in11)
  [adc_o11] -> pfb_fir_real/13(pol1_in12)
  [adc_o12] -> pfb_fir_real/14(pol1_in13)
  [adc_o13] -> pfb_fir_real/15(pol1_in14)
  [adc_o14] -> pfb_fir_real/16(pol1_in15)
  [adc_o15] -> pfb_fir_real/17(pol1_in16)
  pfb_fir_real/1(sync_out) -> [pfb_sync]
  pfb_fir_real/2(pol1_out1) -> [pfb_o00]
  pfb_fir_real/3(pol1_out2) -> [pfb_o01]
  pfb_fir_real/4(pol1_out3) -> [pfb_o02]
  pfb_fir_real/5(pol1_out4) -> [pfb_o03]
  pfb_fir_real/6(pol1_out5) -> [pfb_o04]
  pfb_fir_real/7(pol1_out6) -> [pfb_o05]
  pfb_fir_real/8(pol1_out7) -> [pfb_o06]
  pfb_fir_real/9(pol1_out8) -> [pfb_o07]
  pfb_fir_real/10(pol1_out9) -> [pfb_o08]
  pfb_fir_real/11(pol1_out10) -> [pfb_o09]
  pfb_fir_real/12(pol1_out11) -> [pfb_o10]
  pfb_fir_real/13(pol1_out12) -> [pfb_o11]
  pfb_fir_real/14(pol1_out13) -> [pfb_o12]
  pfb_fir_real/15(pol1_out14) -> [pfb_o13]
  pfb_fir_real/16(pol1_out15) -> [pfb_o14]
  default_fft_shift/1 -> fft_shift/1
  fft_shift/1 -> slice_fft_shift/1
  equalizer/2 -> [eq_out0]
  equalizer/3 -> [eq_out1]
  equalizer/4 -> [eq_out2]
  equalizer/5 -> [eq_out3]
  equalizer/6 -> [eq_out4]
  equalizer/7 -> [eq_out5]
  equalizer/8 -> [eq_out6]
  equalizer/9 -> [eq_out7]
  [fft_sync] -> equalizer/1
  [fft_out0] -> equalizer/2
  [fft_out1] -> equalizer/3
  [fft_out2] -> equalizer/4
  [fft_out3] -> equalizer/5
  [fft_out4] -> equalizer/6
  [fft_out5] -> equalizer/7
  [fft_out6] -> equalizer/8
  [fft_out7] -> equalizer/9
  fft_wideband_real/1(sync_out) -> [fft_sync]
  [ext_led3] -> Delay8/1
  Concat/1 -> status/1
  status/1 -> Terminator8/1
  Delay2/1 -> [fft_shift]
  Delay3/1 -> [eq_coeff]
  [fft_shift] -> fft_wideband_real/2(shift)
  slice_fft_shift/1 -> Delay2/1
  Delay5/1 -> led_sync/1
  Delay6/1 -> led_armed/1
  Delay7/1 -> led_acquire/1
  Delay8/1 -> led_adc_oor/1
  control15/1 -> [control15]
  control14/1 -> [control14]
  control13/1 -> [control13]
  control12/1 -> [control12]
  control11/1 -> [control11]
  control10/1 -> [control10]
  control9/1 -> [control09]
  control8/1 -> [control08]
  control7/1 -> [control07]
  control6/1 -> [control06]
  control5/1 -> [control05]
  control4/1 -> [capt_reset]
  control3/1 -> [acquire]
  control2/1 -> [fifo_reset]
  control1/1 -> [force_trig]
  control0/1 -> [arm_reset]
  [capt_reset] -> capt_fft/11
  [acquire] -> capt_fft/9
  [fft_sync] -> capt_fft/10
  adc083000x2/17(adc0_sync) -> adc_ctrl/1
  adc083000x2/18(adc0_outofrange) -> adc_ctrl/2
  adc083000x2/19(adc0_data_valid) -> adc_ctrl/3
  adc083000x2/20(adc1_sync) -> adc_ctrl/4
  adc083000x2/21(adc1_outofrange) -> adc_ctrl/5
  equalizer/1 -> [eq_sync]
  adc083000x2/22(adc1_data_valid) -> adc_ctrl/6
  sim_sync0/1 -> adc083000x2/2(adc0_sync_sim)
  sim_oor0/1 -> adc083000x2/3(adc0_outofrange_sim)
  sim_valid0/1 -> adc083000x2/4(adc0_data_valid_sim)
  sim_sync1/1 -> adc083000x2/5(adc1_sync_sim)
  sim_oor1/1 -> adc083000x2/6(adc1_outofrange_sim)
  sim_valid1/1 -> adc083000x2/7(adc1_data_valid_sim)
  [pfb_o00] -> downshift/1
  [pfb_o01] -> downshift/2
  [pfb_o02] -> downshift/3
  [pfb_o03] -> downshift/4
  [pfb_o04] -> downshift/5
  [pfb_o05] -> downshift/6
  [pfb_o06] -> downshift/7
  [pfb_o07] -> downshift/8
  [pfb_o08] -> downshift/9
  [pfb_o09] -> downshift/10
  [pfb_o10] -> downshift/11
  [pfb_o11] -> downshift/12
  [pfb_o12] -> downshift/13
  [pfb_o13] -> downshift/14
  [pfb_o14] -> downshift/15
  [pfb_o15] -> downshift/16
  downshift/1 -> fft_wideband_real/3(in0)
  downshift/2 -> fft_wideband_real/4(in1)
  downshift/3 -> fft_wideband_real/5(in2)
  downshift/4 -> fft_wideband_real/6(in3)
  downshift/5 -> fft_wideband_real/7(in4)
  downshift/6 -> fft_wideband_real/8(in5)
  downshift/7 -> fft_wideband_real/9(in6)
  downshift/8 -> fft_wideband_real/10(in7)
  downshift/9 -> fft_wideband_real/11(in8)
  downshift/10 -> fft_wideband_real/12(in9)
  downshift/11 -> fft_wideband_real/13(in10)
  downshift/12 -> fft_wideband_real/14(in11)
  downshift/13 -> fft_wideband_real/15(in12)
  downshift/14 -> fft_wideband_real/16(in13)
  downshift/15 -> fft_wideband_real/17(in14)
  downshift/16 -> fft_wideband_real/18(in15)
  pfb_fir_real/17(pol1_out16) -> [pfb_o15]
  [eq_coeff] -> equalizer/10
  equalizer/10 -> [eq_clip]
  fft_wideband_real/10(of) -> [fft_of]
  [arm_reset] -> armed_trig/2
  [force_trig] -> Logical1/2
  armed_trig/1 -> sync_gen2/1(rst)
  armed_trig/2 -> [armed]
  Logical1/1 -> armed_trig/1
  sync_gen2/1(sync) -> [gen_sync]
  [tx_sync] -> fake_xaui/1
  [tx_dataX] -> fake_xaui/2
  [tx_valid] -> fake_xaui/3
  [fifo_reset] -> xaui0/2
  [tx_dataY] -> xaui0/3
  [tx_valid] -> xaui0/5
  [fifo_reset] -> xaui1/2
  [tx_dataZ] -> xaui1/3
  [tx_oob] -> xaui1/4
  [tx_valid] -> xaui1/5
  xaui0/1 -> [rx_dataY]
  xaui0/2 -> [rx_oobY]
  xaui0/3 -> Terminator3/1
  xaui0/4 -> [rx_validY]
  xaui0/5 -> Terminator4/1
  xaui0/6 -> Terminator9/1
  xaui0/7 -> Terminator2/1
  xaui1/1 -> [rx_dataZ]
  xaui1/2 -> [rx_oobZ]
  xaui1/3 -> Terminator12/1
  xaui1/4 -> [rx_validZ]
  xaui1/5 -> Terminator14/1
  xaui1/6 -> Terminator15/1
  xaui1/7 -> Terminator11/1
  fake_xaui/2 -> [rx_dataX]
  fake_xaui/3 -> [rx_validX]
  fake_xaui/1 -> [syncX]
  [tx_valid] -> xaui0/1
  [tx_valid] -> xaui1/1
  [eq_out0] -> clump/2
  [eq_out1] -> clump/3
  [eq_out2] -> clump/4
  [eq_out3] -> clump/5
  [eq_out4] -> clump/6
  [eq_out5] -> clump/7
  [eq_out6] -> clump/8
  [eq_out7] -> clump/9
  Concat1/1 -> [tx_oob]
  Constant12/1 -> Concat1/1
  [tx_sync] -> Concat1/2
  [tx_oob] -> xaui0/4
  [rx_oobZ] -> control25/1
  [rx_oobY] -> control24/1
  control24/1 -> [syncY]
  control25/1 -> [syncZ]
  [syncX] -> xaui_resync/1
  [rx_dataX] -> xaui_resync/2
  [rx_validX] -> xaui_resync/3
  [syncY] -> xaui_resync/4
  [rx_dataY] -> xaui_resync/5
  [rx_validY] -> xaui_resync/6
  [syncZ] -> xaui_resync/7
  [rx_dataZ] -> xaui_resync/8
  [rx_validZ] -> xaui_resync/9
  xaui_resync/1 -> [xr_sync]
  xaui_resync/2 -> [xr_X]
  xaui_resync/3 -> [xr_Y]
  xaui_resync/4 -> [xr_Z]
  xaui_resync/5 -> [xr_valid]
  [xr_sync] -> unclump/1
  [xr_X] -> unclump/2
  [xr_Y] -> unclump/3
  [xr_Z] -> unclump/4
  unclump/1 -> [uc_sync]
  unclump/2 -> [XA]
  unclump/3 -> [XB]
  unclump/4 -> [XC]
  unclump/5 -> [YA]
  unclump/6 -> [YB]
  unclump/7 -> [YC]
  unclump/8 -> [ZA]
  unclump/9 -> [ZB]
  unclump/10 -> [ZC]
  cross_mult_A/1 -> [cmA_sync]
  cross_mult_A/2 -> [A_XX_r]
  cross_mult_A/3 -> [A_YY_r]
  cross_mult_A/4 -> [A_ZZ_r]
  cross_mult_A/5 -> [A_XY_r]
  cross_mult_A/6 -> [A_XY_i]
  cross_mult_A/7 -> [A_YZ_r]
  cross_mult_A/8 -> [A_YZ_i]
  cross_mult_A/9 -> [A_ZX_r]
  cross_mult_A/10 -> [A_ZX_i]
  [uc_sync] -> cross_mult_A/1
  [XA] -> cross_mult_A/2
  [YA] -> cross_mult_A/3
  [ZA] -> cross_mult_A/4
  [cmA_sync] -> vacc_A/1
  [A_XX_r] -> vacc_A/2
  [A_YY_r] -> vacc_A/3
  [A_ZZ_r] -> vacc_A/4
  [A_XY_r] -> vacc_A/5
  [A_XY_i] -> vacc_A/6
  [A_YZ_r] -> vacc_A/7
  [A_YZ_i] -> vacc_A/8
  [A_ZX_r] -> vacc_A/9
  [A_ZX_i] -> vacc_A/10
  [vaccA_XX_r] -> capt_auto_acc_A/1
  [vaccA_YY_r] -> capt_auto_acc_A/2
  [vaccA_ZZ_r] -> capt_auto_acc_A/3
  [vaccA_sync] -> capt_auto_acc_A/5
  [vaccA_sync] -> capt_imag_acc_A/5
  [vaccA_XY_r] -> capt_real_acc_A/1
  [vaccA_YZ_r] -> capt_real_acc_A/2
  [vaccA_ZX_r] -> capt_real_acc_A/3
  [vaccA_sync] -> capt_real_acc_A/5
  [vaccA_XY_i] -> capt_imag_acc_A/1
  [vaccA_YZ_i] -> capt_imag_acc_A/2
  [vaccA_ZX_i] -> capt_imag_acc_A/3
  [acquire] -> capt_auto_acc_A/4
  [capt_reset] -> capt_auto_acc_A/6
  [acquire] -> capt_real_acc_A/4
  [capt_reset] -> capt_real_acc_A/6
  [acquire] -> capt_imag_acc_A/4
  [capt_reset] -> capt_imag_acc_A/6
  vacc_A/1 -> [vaccA_sync]
  vacc_A/2 -> [vaccA_XX_r]
  vacc_A/3 -> [vaccA_YY_r]
  vacc_A/4 -> [vaccA_ZZ_r]
  vacc_A/5 -> [vaccA_XY_r]
  vacc_A/6 -> [vaccA_XY_i]
  vacc_A/7 -> [vaccA_YZ_r]
  vacc_A/8 -> [vaccA_YZ_i]
  vacc_A/9 -> [vaccA_ZX_r]
  vacc_A/10 -> [vaccA_ZX_i]
  vacc_A/11 -> [vaccA_valid]
  shuffle/1 -> [tx_sync]
  shuffle/2 -> [tx_dataX]
  shuffle/3 -> [tx_dataY]
  shuffle/4 -> [tx_dataZ]
  shuffle/5 -> [tx_valid]
  [eq_sync] -> clump/1
  clump/1 -> shuffle/1
  clump/2 -> shuffle/2
  clump/3 -> shuffle/3
  clump/4 -> shuffle/4
  clump/5 -> shuffle/5
  default_corr_id/1 -> corr_id/1
  corr_id/1 -> slice_corr_id/1
  Delay1/1 -> [corr_id]
  slice_corr_id/1 -> Delay1/1
  [corr_id] -> shuffle/6
  cross_mult_B/1 -> [cmB_sync]
  cross_mult_B/2 -> [B_XX_r]
  cross_mult_B/3 -> [B_YY_r]
  cross_mult_B/4 -> [B_ZZ_r]
  cross_mult_B/5 -> [B_XY_r]
  cross_mult_B/6 -> [B_XY_i]
  cross_mult_B/7 -> [B_YZ_r]
  cross_mult_B/8 -> [B_YZ_i]
  cross_mult_B/9 -> [B_ZX_r]
  cross_mult_B/10 -> [B_ZX_i]
  [uc_sync] -> cross_mult_B/1
  [XB] -> cross_mult_B/2
  [YB] -> cross_mult_B/3
  [ZB] -> cross_mult_B/4
  [cmB_sync] -> vacc_B/1
  [B_XX_r] -> vacc_B/2
  [B_YY_r] -> vacc_B/3
  [B_ZZ_r] -> vacc_B/4
  [B_XY_r] -> vacc_B/5
  [B_XY_i] -> vacc_B/6
  [B_YZ_r] -> vacc_B/7
  [B_YZ_i] -> vacc_B/8
  [B_ZX_r] -> vacc_B/9
  [B_ZX_i] -> vacc_B/10
  vacc_B/1 -> [vaccB_sync]
  vacc_B/2 -> [vaccB_XX_r]
  vacc_B/3 -> [vaccB_YY_r]
  vacc_B/4 -> [vaccB_ZZ_r]
  vacc_B/5 -> [vaccB_XY_r]
  vacc_B/6 -> [vaccB_XY_i]
  vacc_B/7 -> [vaccB_YZ_r]
  vacc_B/8 -> [vaccB_YZ_i]
  vacc_B/9 -> [vaccB_ZX_r]
  vacc_B/10 -> [vaccB_ZX_i]
  vacc_B/11 -> [vaccB_valid]
  cross_mult_C/1 -> [cmC_sync]
  cross_mult_C/2 -> [C_XX_r]
  cross_mult_C/3 -> [C_YY_r]
  cross_mult_C/4 -> [C_ZZ_r]
  cross_mult_C/5 -> [C_XY_r]
  cross_mult_C/6 -> [C_XY_i]
  cross_mult_C/7 -> [C_YZ_r]
  cross_mult_C/8 -> [C_YZ_i]
  cross_mult_C/9 -> [C_ZX_r]
  cross_mult_C/10 -> [C_ZX_i]
  [uc_sync] -> cross_mult_C/1
  [XC] -> cross_mult_C/2
  [YC] -> cross_mult_C/3
  [ZC] -> cross_mult_C/4
  [cmC_sync] -> vacc_C/1
  [C_XX_r] -> vacc_C/2
  [C_YY_r] -> vacc_C/3
  [C_ZZ_r] -> vacc_C/4
  [C_XY_r] -> vacc_C/5
  [C_XY_i] -> vacc_C/6
  [C_YZ_r] -> vacc_C/7
  [C_YZ_i] -> vacc_C/8
  [C_ZX_r] -> vacc_C/9
  [C_ZX_i] -> vacc_C/10
  vacc_C/1 -> [vaccC_sync]
  vacc_C/2 -> [vaccC_XX_r]
  vacc_C/3 -> [vaccC_YY_r]
  vacc_C/4 -> [vaccC_ZZ_r]
  vacc_C/5 -> [vaccC_XY_r]
  vacc_C/6 -> [vaccC_XY_i]
  vacc_C/7 -> [vaccC_YZ_r]
  vacc_C/8 -> [vaccC_YZ_i]
  vacc_C/9 -> [vaccC_ZX_r]
  vacc_C/10 -> [vaccC_ZX_i]
  vacc_C/11 -> [vaccC_valid]
  [vaccB_XX_r] -> capt_auto_acc_B/1
  [vaccB_YY_r] -> capt_auto_acc_B/2
  [vaccB_ZZ_r] -> capt_auto_acc_B/3
  [vaccB_sync] -> capt_auto_acc_B/5
  [vaccB_sync] -> capt_imag_acc_B/5
  [vaccB_XY_r] -> capt_real_acc_B/1
  [vaccB_YZ_r] -> capt_real_acc_B/2
  [vaccB_ZX_r] -> capt_real_acc_B/3
  [vaccB_sync] -> capt_real_acc_B/5
  [vaccB_XY_i] -> capt_imag_acc_B/1
  [vaccB_YZ_i] -> capt_imag_acc_B/2
  [vaccB_ZX_i] -> capt_imag_acc_B/3
  [acquire] -> capt_auto_acc_B/4
  [capt_reset] -> capt_auto_acc_B/6
  [acquire] -> capt_real_acc_B/4
  [capt_reset] -> capt_real_acc_B/6
  [acquire] -> capt_imag_acc_B/4
  [capt_reset] -> capt_imag_acc_B/6
  [vaccC_XX_r] -> capt_auto_acc_C/1
  [vaccC_YY_r] -> capt_auto_acc_C/2
  [vaccC_ZZ_r] -> capt_auto_acc_C/3
  [vaccC_sync] -> capt_auto_acc_C/5
  [vaccC_sync] -> capt_imag_acc_C/5
  [vaccC_XY_r] -> capt_real_acc_C/1
  [vaccC_YZ_r] -> capt_real_acc_C/2
  [vaccC_ZX_r] -> capt_real_acc_C/3
  [vaccC_sync] -> capt_real_acc_C/5
  [vaccC_XY_i] -> capt_imag_acc_C/1
  [vaccC_YZ_i] -> capt_imag_acc_C/2
  [vaccC_ZX_i] -> capt_imag_acc_C/3
  [acquire] -> capt_auto_acc_C/4
  [capt_reset] -> capt_auto_acc_C/6
  [acquire] -> capt_real_acc_C/4
  [capt_reset] -> capt_real_acc_C/6
  [acquire] -> capt_imag_acc_C/4
  [capt_reset] -> capt_imag_acc_C/6
  Delay9/1 -> control15/1, control14/1, control13/1, control12/1, control11/1, control10/1, control9/1, control8/1, control7/1, control6/1, control5/1, control4/1, control3/1, control2/1, control1/1, control0/1
  control/1 -> Delay9/1
  [eq_out0] -> capt_eq/1
  [eq_out1] -> capt_eq/2
  [eq_out2] -> capt_eq/3
  [eq_out3] -> capt_eq/4
  [eq_out4] -> capt_eq/5
  [eq_out5] -> capt_eq/6
  [eq_out6] -> capt_eq/7
  [eq_out7] -> capt_eq/8
  [capt_reset] -> capt_eq/11
  [acquire] -> capt_eq/9
  [eq_sync] -> capt_eq/10
  adc_ctrl/1 -> [adc_sync]
  adc_ctrl/2 -> [adc_oor]
  adc_ctrl/3 -> [adc_valid]
  [adc_sync] -> Logical1/1
  pulse_ext3/1 -> Concat/13
  pulse_ext2/1 -> Concat/14, [ext_led2]
  pulse_ext1/1 -> Concat/15, [ext_led1]
  pulse_ext0/1 -> Concat/16, [ext_led0]
  adc083000x2/1(adc0_s0) -> Terminator10/1
  adc083000x2/2(adc1_s0) -> Terminator13/1
  adc083000x2/3(adc0_s1) -> Terminator16/1
  adc083000x2/4(adc1_s1) -> Terminator17/1
  adc083000x2/5(adc0_s2) -> Terminator18/1
  adc083000x2/6(adc1_s2) -> Terminator19/1
  adc083000x2/7(adc0_s3) -> Terminator20/1
  adc083000x2/8(adc1_s3) -> Terminator21/1
  adc083000x2/9(adc0_s4) -> Terminator22/1
  adc083000x2/10(adc1_s4) -> Terminator23/1
  adc083000x2/11(adc0_s5) -> Terminator24/1
  adc083000x2/12(adc1_s5) -> Terminator25/1
  adc083000x2/13(adc0_s6) -> Terminator26/1
  adc083000x2/14(adc1_s6) -> Terminator27/1
  adc083000x2/15(adc0_s7) -> Terminator28/1
  adc083000x2/16(adc1_s7) -> Terminator29/1
  adc_tvg/17 -> [adc_o15]
  adc_tvg/16 -> [adc_o14]
  adc_tvg/15 -> [adc_o13]
  adc_tvg/14 -> [adc_o12]
  adc_tvg/13 -> [adc_o11]
  adc_tvg/12 -> [adc_o10]
  adc_tvg/11 -> [adc_o09]
  adc_tvg/10 -> [adc_o08]
  adc_tvg/9 -> [adc_o07]
  adc_tvg/8 -> [adc_o06]
  adc_tvg/7 -> [adc_o05]
  adc_tvg/6 -> [adc_o04]
  adc_tvg/5 -> [adc_o03]
  adc_tvg/4 -> [adc_o02]
  adc_tvg/3 -> [adc_o01]
  adc_tvg/2 -> [adc_o00]
  adc_tvg/1 -> [tvg_sync]
  [gen_sync] -> adc_tvg/1
  [adc_o00] -> capt_adc/1
  [adc_o01] -> capt_adc/2
  [adc_o02] -> capt_adc/3
  [adc_o03] -> capt_adc/4
  [adc_o04] -> capt_adc/5
  [adc_o05] -> capt_adc/6
  [adc_o06] -> capt_adc/7
  [adc_o07] -> capt_adc/8
  [adc_o08] -> capt_adc/9
  [adc_o09] -> capt_adc/10
  [adc_o10] -> capt_adc/11
  [adc_o11] -> capt_adc/12
  [adc_o12] -> capt_adc/13
  [adc_o13] -> capt_adc/14
  [adc_o14] -> capt_adc/15
  [adc_o15] -> capt_adc/16
  [acquire] -> capt_adc/17
  [tvg_sync] -> capt_adc/18
  [capt_reset] -> capt_adc/19
